simulink model "leda_actual_1s"  (Simulink 7.4)
settings: Solver=ode45  StartTime=0.0  StopTime=50000  RelTol=1e-3
undefined symbols (not defined in the file): pfb_coeff_gen_calc
libraries: xbsIndex_r4 x5755, casper_library_misc x176, casper_library_delays x48, xps_library x39, xbsReplacements_r4 x4, casper_library_ffts_twiddle x2, simulink x1
(part 1 of 84)
settings Model: Creator=jkocz  LastModifiedBy=jkocz

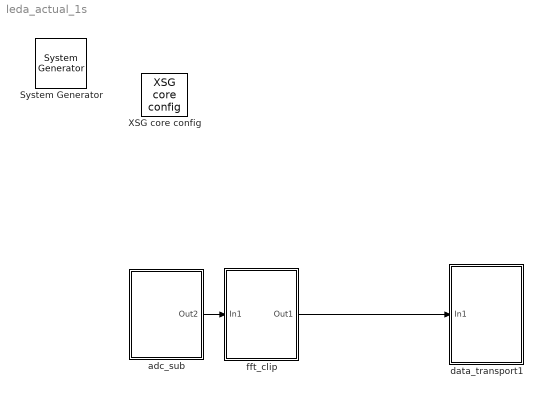
== system leda_actual_1s ==
  " System Generator" : xbsIndex_r4/ System Generator  Tag=genX  SystemSampleTime=-1  xilinxfamily=virtex5  part=xc5vsx95t  speed=-1  package=ff1136  synthesis_tool=XST  clock_wrapper=Clock Enables  directory=./leda_4096_32_test/sysgen  testbench=off  simulink_period=1  sysclk_period=5  dcm_input_clock_period=100  incr_netlist=off  trim_vbits=Everywhere in SubSystem  dbl_ovrd=According to Block Masks  core_generation=According to Block Masks  run_coregen=off  deprecated_control=off  eval_field=0  block_type=sysgen
      settings: block_version=11.4
  XSG core config : xps_library/XSG core config  Tag=xps:xsg  SystemSampleTime=-1  hw_sys=ROACH2:sx475t  clk_src=aux_clk  ROACH_clk_src=adc0_clk  ROACH2_clk_src=aux_clk  clk_rate=200  sample_period=1  synthesis_tool=XST
  adc_sub : Subsystem
  data_transport1 : Subsystem
  fft_clip : Subsystem
  wires:
  fft_clip/1(Out1) -> data_transport1/1(In1)
  adc_sub/1(Out2) -> fft_clip/1(In1)

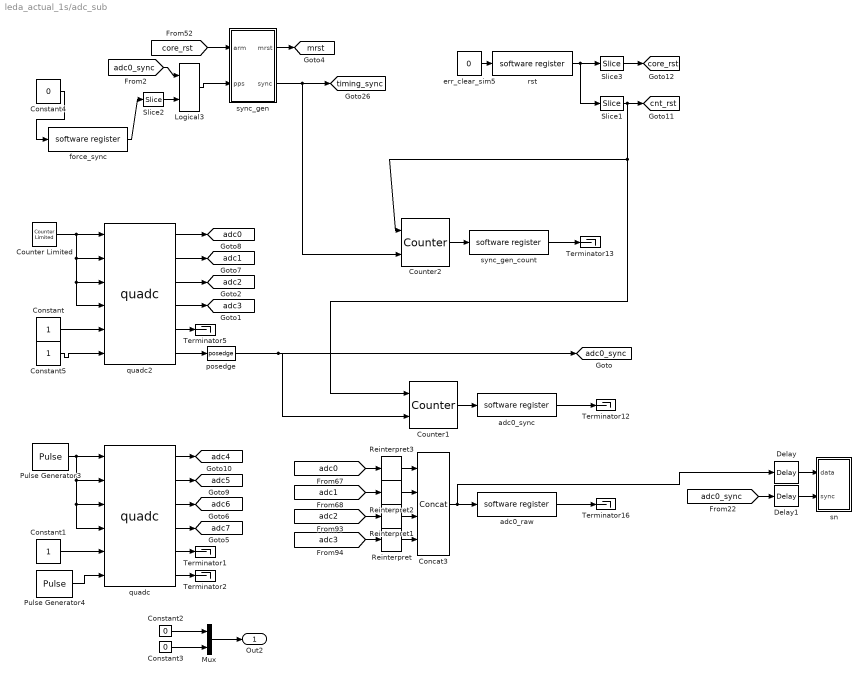
== system leda_actual_1s/adc_sub ==
  Concat3 : xbsIndex_r4/Concat  num_inputs=4  dbl_ovrd=off  block_type=concat
      settings: block_version=10.1.3
  Constant : Constant  OutDataType=sfix(16)  OutScaling=2^0  SampleTime=1/4
  Constant1 : Constant  OutDataType=sfix(16)  OutScaling=2^0  SampleTime=1/4
  Constant2 : Constant  Value=0
  Constant3 : Constant  Value=0
  Constant4 : Constant  Value=0  OutDataType=sfix(16)  OutScaling=2^0  SampleTime=1/4
  Constant5 : Constant  OutDataType=sfix(16)  OutScaling=2^0  SampleTime=1/4
  Counter Limited : simulink/Sources/Counter Limited  SystemSampleTime=-1  uplimit=4095  tsamp=-1
  Counter1 : xbsIndex_r4/Counter  cnt_type=Free Running  cnt_to=Inf  operation=Up  start_count=0  cnt_by_val=1  arith_type=Unsigned  n_bits=64  bin_pt=0  load_pin=off  rst=on  en=on  explicit_period=on  period=1  dbl_ovrd=off  use_behavioral_HDL=off  implementation=Fabric  block_type=counter
      settings: block_version=11.4
  Counter2 : xbsIndex_r4/Counter  cnt_type=Free Running  cnt_to=Inf  operation=Up  start_count=0  cnt_by_val=1  arith_type=Unsigned  n_bits=64  bin_pt=0  load_pin=off  rst=on  en=on  explicit_period=on  period=1  dbl_ovrd=off  use_behavioral_HDL=off  implementation=Fabric  block_type=counter
      settings: block_version=11.4
  Delay : xbsIndex_r4/Delay  en=off  latency=2  dbl_ovrd=off  reg_retiming=off  block_type=delay
      settings: block_version=11.4
  Delay1 : xbsIndex_r4/Delay  en=off  latency=5  dbl_ovrd=off  reg_retiming=off  block_type=delay
      settings: block_version=11.4
  Logical3 : xbsIndex_r4/Logical  logical_function=OR  inputs=2  en=off  latency=0  precision=Full  arith_type=Unsigned  n_bits=16  bin_pt=0  align_bp=on  dbl_ovrd=off  block_type=logical
  Mux : Mux  Inputs=2
  Pulse Generator3 : DiscretePulseGenerator  Amplitude=0.75  Period=2^4  PulseWidth=1  PhaseDelay=9+13+2^8  SampleTime=1/4
  Pulse Generator4 : DiscretePulseGenerator  Amplitude=1  Period=2^16  PulseWidth=1  PhaseDelay=0  SampleTime=1
  Reinterpret{,1..3} (4 alike) : xbsIndex_r4/Reinterpret  force_arith_type=on  arith_type=Unsigned  force_bin_pt=on  bin_pt=0  block_type=reinterpret
      settings: infoedit="Changes signal type without altering the binary representation.   You can changed the signal between signed and unsigned, and relocate the binary point.<br><br>Hardware notes: In hardware this block costs nothing.<br><br>Example:  Suppose the input is 6 bits wide, signed, with 2 fractional bits, and the output is forced to unsigned with 0 fractional bits.  Then an input of -2.0 (1110.00 in binary 2's complement) becomes an output of 56 (111000 in binary)."
  Slice1 : xbsIndex_r4/Slice  nbits=1  boolean_output=on  mode=Lower Bit Location + Width  bit1=0  base1=MSB of Input  bit0=0  base0=LSB of Input  dbl_ovrd=off  block_type=slice
      settings: block_version=10.1.3
  Slice2 : xbsIndex_r4/Slice  nbits=1  boolean_output=on  mode=Lower Bit Location + Width  bit1=0  base1=MSB of Input  bit0=0  base0=LSB of Input  dbl_ovrd=off  block_type=slice
      settings: block_version=10.1.3
  Slice3 : xbsIndex_r4/Slice  nbits=1  boolean_output=on  mode=Lower Bit Location + Width  bit1=0  base1=MSB of Input  bit0=1  base0=LSB of Input  dbl_ovrd=off  block_type=slice
      settings: block_version=10.1.3
  Terminator{1..2,5,12..13,16} (6 alike) : Terminator
  adc0_raw : xps_library/software register  Tag=xps:sw_reg  SystemSampleTime=-1  io_dir=To Processor  arith_type=Unsigned  bitwidth=32  bin_pt=0  sample_period=1  gw_name=leda_actual_1s_adc_sub_adc0_raw_user_data_in
  adc0_sync : xps_library/software register  Tag=xps:sw_reg  SystemSampleTime=-1  io_dir=To Processor  arith_type=Unsigned  bitwidth=32  bin_pt=0  sample_period=1  gw_name=leda_actual_1s_adc_sub_adc0_sync_user_data_in
  err_clear_sim5 : Constant  Value=0  OutDataType=sfix(16)  OutScaling=2^0
  force_sync : xps_library/software register  Tag=xps:sw_reg  SystemSampleTime=-1  io_dir=From Processor  arith_type=Unsigned  bitwidth=32  bin_pt=0  sample_period=1  gw_name=leda_actual_1s_adc_sub_force_sync_user_data_out
      link override r_10gbe_demo_gbe_core_rst_user_data_out: arith_type=Unsigned  n_bits=32  bin_pt=0  period=1
      link override r_10gbe_test_cnt_rst_user_data_out: arith_type=Unsigned  n_bits=32  bin_pt=0  period=1
      link override r_10gbe_test_gbe_core_rst_user_data_out: arith_type=Unsigned  n_bits=32  bin_pt=0  period=1
      link override r_xaui_test_cnt_rst_user_data_out: arith_type=Unsigned  n_bits=32  bin_pt=0  period=1
  posedge : casper_library_misc/posedge  Tag=Rising Edge Detector  SystemSampleTime=-1
  quadc : xps_library/quadc  Tag=xps:quadc  SystemSampleTime=-1  adc_brd=0  adc_clk_rate=200
  quadc2 : xps_library/quadc  Tag=xps:quadc  SystemSampleTime=-1  adc_brd=0  adc_clk_rate=200
  rst : xps_library/software register  Tag=xps:sw_reg  SystemSampleTime=-1  io_dir=From Processor  arith_type=Unsigned  bitwidth=32  bin_pt=0  sample_period=1  gw_name=leda_actual_1s_adc_sub_rst_user_data_out
      link override r_10gbe_demo_gbe_core_rst_user_data_out: arith_type=Unsigned  n_bits=32  bin_pt=0  period=1
      link override r_10gbe_test_cnt_rst_user_data_out: arith_type=Unsigned  n_bits=32  bin_pt=0  period=1
      link override r_10gbe_test_gbe_core_rst_user_data_out: arith_type=Unsigned  n_bits=32  bin_pt=0  period=1
      link override r_xaui_test_cnt_rst_user_data_out: arith_type=Unsigned  n_bits=32  bin_pt=0  period=1
  sn : Subsystem
  sync_gen : Subsystem  mask(sync_gen_hw){sync_period_bits=27}
      mask prompts: sync_period_bits "Size of sync generator counter (bits)"
      mask help:
      | This circuit implements a scheme for synchronizing a design to within an FPGA clock of an external 1 pulse-per-second signal.
      |
      | * An arm pulse will cause the sync_gen to stop issuing sync pulses.
      | * It will then ignore the next input trigger (1PPS), while sync is flushed through the DSP pipeline.
      | * It will then lock onto the next trigger (1PPS) and issue syncs thereafter every sync_period clocks.
      | * You will need a multiple of (pfb_fir taps*LCM(FFT reorder orders) *fft bins/parallel inputs). See the sync memo for further details.
  sync_gen_count : xps_library/software register  Tag=xps:sw_reg  SystemSampleTime=-1  io_dir=To Processor  arith_type=Unsigned  bitwidth=32  bin_pt=0  sample_period=1  gw_name=leda_actual_1s_adc_sub_sync_gen_count_user_data_in
  Out2 : Outport  OutDataType=sfix(16)  OutScaling=2^0
  tag scope: adc0=global, adc0_sync=global, adc1=global, adc2=global, adc3=global, adc4=global, adc5=global, adc6=global, adc7=global, cnt_rst=global, core_rst=global, mrst=global, timing_sync=global
  wires:
  sync_gen/1(mrst) -> [mrst]
  sync_gen/2(sync) -> [timing_sync], Counter2/2
  Logical3/1 -> sync_gen/2(pps)
  [core_rst] -> sync_gen/1(arm)
  quadc2/1 -> [adc0]
  Counter Limited/1 -> quadc2/4, quadc2/3, quadc2/2, quadc2/1
  quadc2/2 -> [adc1]
  quadc2/3 -> [adc2]
  quadc2/4 -> [adc3]
  quadc2/5 -> Terminator5/1
  quadc2/6 -> posedge/1
  Constant/1 -> quadc2/5
  quadc/1 -> [adc4]
  Pulse Generator3/1 -> quadc/4, quadc/3, quadc/2, quadc/1
  quadc/2 -> [adc5]
  quadc/3 -> [adc6]
  quadc/4 -> [adc7]
  quadc/5 -> Terminator1/1
  quadc/6 -> Terminator2/1
  Constant1/1 -> quadc/5
  Pulse Generator4/1 -> quadc/6
  [adc0_sync] -> Logical3/1
  Mux/1 -> Out2/1
  Constant2/1 -> Mux/1
  Constant3/1 -> Mux/2
  err_clear_sim5/1 -> rst/1
  rst/1 -> Slice3/1, Slice1/1
  Slice1/1 -> [cnt_rst], Counter1/1, Counter2/1
  Slice3/1 -> [core_rst]
  Constant5/1 -> quadc2/6
  force_sync/1 -> Slice2/1
  Slice2/1 -> Logical3/2
  Constant4/1 -> force_sync/1
  adc0_raw/1 -> Terminator16/1
  adc0_sync/1 -> Terminator12/1
  Counter1/1 -> adc0_sync/1
  sync_gen_count/1 -> Terminator13/1
  Counter2/1 -> sync_gen_count/1
  Concat3/1 -> adc0_raw/1, Delay/1
  [adc0] -> Reinterpret3/1
  [adc1] -> Reinterpret2/1
  [adc2] -> Reinterpret1/1
  [adc3] -> Reinterpret/1
  Reinterpret3/1 -> Concat3/1
  Reinterpret2/1 -> Concat3/2
  Reinterpret1/1 -> Concat3/3
  Reinterpret/1 -> Concat3/4
  posedge/1 -> Counter1/2, [adc0_sync]
  [adc0_sync] -> Delay1/1
  Delay1/1 -> sn/2(sync)
  Delay/1 -> sn/1(data)

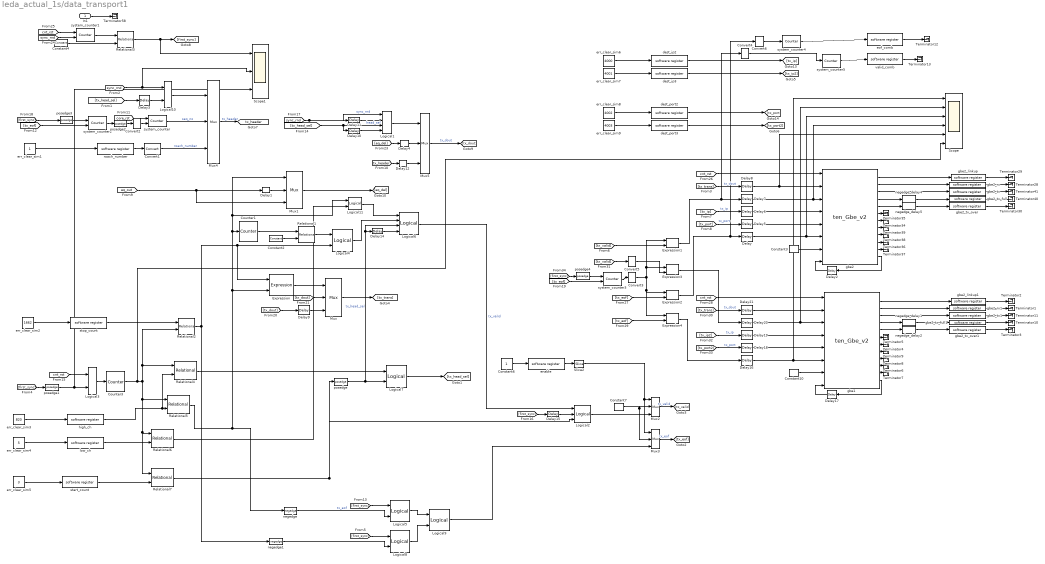
== system leda_actual_1s/data_transport1 ==
  In1 : Inport  OutDataType=sfix(16)  OutScaling=2^0
  Constant10 : xbsIndex_r4/Constant  arith_type=Boolean  const=1  n_bits=1  bin_pt=0  explicit_period=on  period=1  equ=P=C  opselect=C  inp2=PCIN>>17  opr=+  inp1=P  carry=CIN  dbl_ovrd=off  block_type=constant
  Constant2 : xbsIndex_r4/Constant  arith_type=Unsigned  const=25  n_bits=11  bin_pt=0  explicit_period=on  period=1  equ=P=C  opselect=C  inp2=PCIN>>17  opr=+  inp1=P  carry=CIN  dbl_ovrd=off  block_type=constant
  Constant3 : xbsIndex_r4/Constant  arith_type=Boolean  const=1  n_bits=1  bin_pt=0  explicit_period=on  period=1  equ=P=C  opselect=C  inp2=PCIN>>17  opr=+  inp1=P  carry=CIN  dbl_ovrd=off  block_type=constant
  Constant4 : xbsIndex_r4/Constant  arith_type=Unsigned  const=1  n_bits=2  bin_pt=0  explicit_period=on  period=1  equ=P=C  opselect=C  inp2=PCIN>>17  opr=+  inp1=P  carry=CIN  dbl_ovrd=off  block_type=constant
  Constant6 : Constant  OutDataType=sfix(16)  OutScaling=2^0
  Constant7 : xbsIndex_r4/Constant  arith_type=Boolean  const=0  n_bits=1  bin_pt=0  explicit_period=on  period=1  equ=P=C  opselect=C  inp2=PCIN>>17  opr=+  inp1=P  carry=CIN  dbl_ovrd=off  block_type=constant
  Convert1 : xbsIndex_r4/Convert  arith_type=Unsigned  n_bits=64  bin_pt=0  quantization=Truncate  overflow=Wrap  latency=0  dbl_ovrd=off  pipeline=off  block_type=convert
      settings: block_version=11.4
  Convert{2..6} (5 alike) : xbsIndex_r4/Convert  arith_type=Boolean  n_bits=64  bin_pt=0  quantization=Truncate  overflow=Wrap  latency=0  dbl_ovrd=off  pipeline=off  block_type=convert
      settings: block_version=11.4
  Counter1 : xbsIndex_r4/Counter  cnt_type=Free Running  cnt_to=1023  operation=Up  start_count=0  cnt_by_val=1  arith_type=Unsigned  n_bits=13  bin_pt=0  load_pin=off  rst=on  en=off  explicit_period=on  period=1  dbl_ovrd=off  use_behavioral_HDL=off  implementation=Fabric  use_rpm=on  block_type=counter
      settings: xl_area=[0, 0, 0, 0, 0, 0, 0]
  Counter3 : xbsIndex_r4/Counter  cnt_type=Free Running  cnt_to=1023  operation=Up  start_count=0  cnt_by_val=1  arith_type=Unsigned  n_bits=13  bin_pt=0  load_pin=off  rst=on  en=off  explicit_period=on  period=1  dbl_ovrd=off  use_behavioral_HDL=off  implementation=Fabric  use_rpm=on  block_type=counter
      settings: xl_area=[0, 0, 0, 0, 0, 0, 0]
  Delay{,3,7,16,20} (5 alike) : xbsIndex_r4/Delay  en=off  latency=0  dbl_ovrd=off  reg_retiming=off  block_type=delay
      settings: block_version=11.4
  Delay1 : xbsIndex_r4/Delay  en=off  latency=2  dbl_ovrd=off  reg_retiming=on  block_type=delay
      settings: xl_area=[0, 0, 0, 0, 0, 0, 0]
  Delay{5..6,8,10..11,14,18..19,21} (9 alike) : xbsIndex_r4/Delay  en=off  latency=1  dbl_ovrd=off  reg_retiming=off  block_type=delay
      settings: block_version=11.4
  Delay12 : xbsIndex_r4/Delay  en=off  latency=1  dbl_ovrd=off  reg_retiming=on  block_type=delay
      settings: xl_area=[0, 0, 0, 0, 0, 0, 0]
  Delay15 : xbsIndex_r4/Delay  en=off  latency=3  dbl_ovrd=off  reg_retiming=off  block_type=delay
      settings: block_version=11.4
  Delay17 : xbsIndex_r4/Delay  en=off  latency=1  dbl_ovrd=off  reg_retiming=on  block_type=delay
  Delay2 : xbsIndex_r4/Delay  en=off  latency=1  dbl_ovrd=off  reg_retiming=on  block_type=delay
  Delay4 : xbsIndex_r4/Delay  en=off  latency=0  dbl_ovrd=off  reg_retiming=on  block_type=delay
      settings: xl_area=[0, 0, 0, 0, 0, 0, 0]
  Delay9 : xbsIndex_r4/Delay  en=off  latency=26  dbl_ovrd=off  reg_retiming=off  block_type=delay
      settings: block_version=11.4
  Expression : xbsIndex_r4/Expression  expression=a & ~b  align_bp=on  en=off  latency=0  precision=Full  arith_type=Unsigned  n_bits=16  bin_pt=0  dbl_ovrd=off  block_type=expr
      settings: block_version=11.4
  Expression1 : xbsIndex_r4/Expression  expression=a & b  align_bp=on  en=off  latency=0  precision=User Defined  arith_type=Unsigned  n_bits=1  bin_pt=0  dbl_ovrd=off  block_type=expr
      settings: block_version=11.4
  Expression2 : xbsIndex_r4/Expression  expression=a & b  align_bp=on  en=off  latency=0  precision=User Defined  arith_type=Unsigned  n_bits=1  bin_pt=0  dbl_ovrd=off  block_type=expr
      settings: block_version=11.4
  Expression3 : xbsIndex_r4/Expression  expression=~a & b  align_bp=on  en=off  latency=0  precision=User Defined  arith_type=Unsigned  n_bits=1  bin_pt=0  dbl_ovrd=off  block_type=expr
      settings: block_version=11.4
  Expression4 : xbsIndex_r4/Expression  expression=~a & b  align_bp=on  en=off  latency=0  precision=User Defined  arith_type=Unsigned  n_bits=1  bin_pt=0  dbl_ovrd=off  block_type=expr
      settings: block_version=11.4
  Logical1 : xbsIndex_r4/Logical  logical_function=OR  inputs=4  en=off  latency=0  precision=Full  arith_type=Unsigned  n_bits=16  bin_pt=0  align_bp=on  dbl_ovrd=off  block_type=logical
  Logical{3,7,9..10} (4 alike) : xbsIndex_r4/Logical  logical_function=OR  inputs=2  en=off  latency=0  precision=Full  arith_type=Unsigned  n_bits=16  bin_pt=0  align_bp=on  dbl_ovrd=off  block_type=logical
      settings: block_version=11.4
  Logical{4..5,8,11} (4 alike) : xbsIndex_r4/Logical  logical_function=AND  inputs=2  en=off  latency=0  precision=Full  arith_type=Unsigned  n_bits=16  bin_pt=0  align_bp=on  dbl_ovrd=off  block_type=logical
      settings: block_version=11.4
  Logical2 : xbsIndex_r4/Logical  logical_function=AND  inputs=3  en=off  latency=0  precision=Full  arith_type=Unsigned  n_bits=16  bin_pt=0  align_bp=on  dbl_ovrd=off  block_type=logical
      settings: block_version=11.4
  Logical6 : xbsIndex_r4/Logical  logical_function=OR  inputs=4  en=off  latency=1  precision=Full  arith_type=Unsigned  n_bits=16  bin_pt=0  align_bp=on  dbl_ovrd=off  block_type=logical
      settings: block_version=11.4
  Mux : xbsIndex_r4/Mux  inputs=2  en=off  latency=1  precision=Full  arith_type=Unsigned  n_bits=16  bin_pt=14  quantization=Truncate  overflow=Wrap  dbl_ovrd=off  block_type=mux
      settings: block_version=11.4
  Mux1 : xbsIndex_r4/Mux  inputs=2  en=off  latency=0  precision=Full  arith_type=Unsigned  n_bits=16  bin_pt=14  quantization=Truncate  overflow=Wrap  dbl_ovrd=off  block_type=mux
      settings: block_version=11.4
  Mux2 : xbsIndex_r4/Mux  inputs=2  en=off  latency=1  precision=Full  arith_type="Signed  (2's comp)"  n_bits=8  bin_pt=2  quantization=Truncate  overflow=Wrap  dbl_ovrd=off  block_type=mux
      settings: xl_area=[0, 0, 0, 0, 0, 0, 0]
  Mux3 : xbsIndex_r4/Mux  inputs=2  en=off  latency=1  precision=Full  arith_type="Signed  (2's comp)"  n_bits=8  bin_pt=2  quantization=Truncate  overflow=Wrap  dbl_ovrd=off  block_type=mux
      settings: xl_area=[0, 0, 0, 0, 0, 0, 0]
  Mux4 : xbsIndex_r4/Mux  inputs=2  en=off  latency=0  precision=Full  arith_type="Signed  (2's comp)"  n_bits=8  bin_pt=2  quantization=Truncate  overflow=Wrap  dbl_ovrd=off  block_type=mux
      settings: xl_area=[0, 0, 0, 0, 0, 0, 0]
  Mux5 : xbsIndex_r4/Mux  inputs=2  en=off  latency=0  precision=Full  arith_type="Signed  (2's comp)"  n_bits=8  bin_pt=2  quantization=Truncate  overflow=Wrap  dbl_ovrd=off  block_type=mux
      settings: xl_area=[0, 0, 0, 0, 0, 0, 0]
  Relational1 : xbsIndex_r4/Relational  mode=a>b  en=off  latency=0  dbl_ovrd=off  block_type=relational
      settings: xl_area=[0, 0, 0, 0, 0, 0, 0]  block_version=10.1.3
  Relational2 : xbsIndex_r4/Relational  mode=a>b  en=off  latency=1  dbl_ovrd=off  block_type=relational
      settings: xl_area=[0, 0, 0, 0, 0, 0, 0]  block_version=10.1.3
  Relational3 : xbsIndex_r4/Relational  mode=a>=b  en=off  latency=0  dbl_ovrd=off  block_type=relational
      settings: xl_area=[0, 0, 0, 0, 0, 0, 0]  block_version=10.1.3
  Relational4 : xbsIndex_r4/Relational  mode=a=b  en=off  latency=1  dbl_ovrd=off  block_type=relational
      settings: xl_area=[0, 0, 0, 0, 0, 0, 0]  block_version=10.1.3
  Relational5 : xbsIndex_r4/Relational  mode=a<b  en=off  latency=1  dbl_ovrd=off  block_type=relational
      settings: xl_area=[0, 0, 0, 0, 0, 0, 0]  block_version=10.1.3
  Relational6 : xbsIndex_r4/Relational  mode=a>b  en=off  latency=1  dbl_ovrd=off  block_type=relational
      settings: xl_area=[0, 0, 0, 0, 0, 0, 0]  block_version=10.1.3
  Relational7 : xbsIndex_r4/Relational  mode=a>b  en=off  latency=1  dbl_ovrd=off  block_type=relational
      settings: xl_area=[0, 0, 0, 0, 0, 0, 0]  block_version=10.1.3
  Scope : Scope  NumInputPorts=6
      settings: YMin=-5~-5~-5~-5~0~840  YMax=5~5~5~5~5~850
  Scope1 : Scope  NumInputPorts=3
      settings: YMin=-5~-5~-5  YMax=5~5~5
  Slice2 : xbsIndex_r4/Slice  nbits=1  boolean_output=off  mode=Lower Bit Location + Width  bit1=1  base1=MSB of Input  bit0=0  base0=LSB of Input  dbl_ovrd=off  block_type=slice
      settings: block_version=10.1.3
  Terminator{1..13,28..30,34..41,58} (25 alike) : Terminator
  dest_ip2 : xps_library/software register  Tag=xps:sw_reg  SystemSampleTime=-1  io_dir=From Processor  arith_type=Unsigned  bitwidth=32  bin_pt=0  sample_period=1  gw_name=leda_actual_1s_data_transport1_dest_ip2_user_data_out
      link override r_10gbe_demo_dest_ip_user_data_out: arith_type=Unsigned  n_bits=32  bin_pt=0  period=1
      link override r_10gbe_test_dest_ip_user_data_out: arith_type=Unsigned  n_bits=32  bin_pt=0  period=1
      link override r_xaui_test_cnt_rst_user_data_out: arith_type=Unsigned  n_bits=32  bin_pt=0  period=1
  dest_ip3 : xps_library/software register  Tag=xps:sw_reg  SystemSampleTime=-1  io_dir=From Processor  arith_type=Unsigned  bitwidth=32  bin_pt=0  sample_period=1  gw_name=leda_actual_1s_data_transport1_dest_ip3_user_data_out
      link override r_10gbe_demo_dest_ip_user_data_out: arith_type=Unsigned  n_bits=32  bin_pt=0  period=1
      link override r_10gbe_test_dest_ip_user_data_out: arith_type=Unsigned  n_bits=32  bin_pt=0  period=1
      link override r_xaui_test_cnt_rst_user_data_out: arith_type=Unsigned  n_bits=32  bin_pt=0  period=1
  dest_port2 : xps_library/software register  Tag=xps:sw_reg  SystemSampleTime=-1  io_dir=From Processor  arith_type=Unsigned  bitwidth=32  bin_pt=0  sample_period=1  gw_name=leda_actual_1s_data_transport1_dest_port2_user_data_out
      link override r_10gbe_demo_dest_port_user_data_out: arith_type=Unsigned  n_bits=32  bin_pt=0  period=1
      link override r_10gbe_test_dest_port_user_data_out: arith_type=Unsigned  n_bits=32  bin_pt=0  period=1
      link override r_xaui_test_cnt_rst_user_data_out: arith_type=Unsigned  n_bits=32  bin_pt=0  period=1
  dest_port3 : xps_library/software register  Tag=xps:sw_reg  SystemSampleTime=-1  io_dir=From Processor  arith_type=Unsigned  bitwidth=32  bin_pt=0  sample_period=1  gw_name=leda_actual_1s_data_transport1_dest_port3_user_data_out
      link override r_10gbe_demo_dest_port_user_data_out: arith_type=Unsigned  n_bits=32  bin_pt=0  period=1
      link override r_10gbe_test_dest_port_user_data_out: arith_type=Unsigned  n_bits=32  bin_pt=0  period=1
      link override r_xaui_test_cnt_rst_user_data_out: arith_type=Unsigned  n_bits=32  bin_pt=0  period=1
  enable : xps_library/software register  Tag=xps:sw_reg  SystemSampleTime=-1  io_dir=From Processor  arith_type=Unsigned  bitwidth=32  bin_pt=0  sample_period=1  gw_name=leda_actual_1s_data_transport1_enable_user_data_out
      link override r_10gbe_demo_pkt_sim_run_user_data_out: arith_type=Unsigned  n_bits=32  bin_pt=0  period=1
      link override r_10gbe_test_pkt_sim_run_user_data_out: arith_type=Unsigned  n_bits=32  bin_pt=0  period=1
      link override test_pkt_buffer_10_1_p_mux_up_run_user_data_out: arith_type=Unsigned  n_bits=32  bin_pt=0  period=1
  eof_comb : xps_library/software register  Tag=xps:sw_reg  SystemSampleTime=-1  io_dir=To Processor  arith_type=Unsigned  bitwidth=32  bin_pt=0  sample_period=1  gw_name=leda_actual_1s_data_transport1_eof_comb_user_data_in
      link override r_10gbe_demo_dest_ip_user_data_out: arith_type=Unsigned  n_bits=32  bin_pt=0  period=1
      link override r_10gbe_test_dest_ip_user_data_out: arith_type=Unsigned  n_bits=32  bin_pt=0  period=1
      link override r_xaui_test_cnt_rst_user_data_out: arith_type=Unsigned  n_bits=32  bin_pt=0  period=1
  err_clear_sim1 : Constant  OutDataType=sfix(16)  OutScaling=2^0
  err_clear_sim2 : Constant  Value=1662  OutDataType=sfix(16)  OutScaling=2^0
  err_clear_sim3 : Constant  Value=820  OutDataType=sfix(16)  OutScaling=2^0
  err_clear_sim4 : Constant  Value=5  OutDataType=sfix(16)  OutScaling=2^0
  err_clear_sim5 : Constant  Value=3  OutDataType=sfix(16)  OutScaling=2^0
  err_clear_sim6 : Constant  Value=4000  OutDataType=sfix(16)  OutScaling=2^0
  err_clear_sim7 : Constant  Value=4001  OutDataType=sfix(16)  OutScaling=2^0
  err_clear_sim8 : Constant  Value=4002  OutDataType=sfix(16)  OutScaling=2^0
  err_clear_sim9 : Constant  Value=4003  OutDataType=sfix(16)  OutScaling=2^0
  gbe1 : xps_library/ten_Gbe_v2  Tag=xps:tengbe_v2  SystemSampleTime=-1  flavour=cx4  slot=1  port_r1=0  port_r2_cx4=0  port_r2_sfpp=0  rx_dist_ram=off  large_frames=on  show_param=on  pre_emph_r2=0.74  pre_emph=3  post_emph_r2=0.18  rxeqmix_r2=7  swing_r2=940  swing=800  fab_en=off  fab_mac=hex2dec('123456789105')  fab_ip=192*(2^24) + 168*(2^16) + 6*(2^8) + 15*(2^0)  fab_udp=10002  fab_gate=192*(2^24) + 168*(2^16) + 6*(2^8) + 0  cpu_rx_en=on  cpu_tx_en=on
  gbe2 : xps_library/ten_Gbe_v2  Tag=xps:tengbe_v2  SystemSampleTime=-1  flavour=cx4  slot=1  port_r1=0  port_r2_cx4=0  port_r2_sfpp=0  rx_dist_ram=off  large_frames=on  show_param=on  pre_emph_r2=0.74  pre_emph=3  post_emph_r2=0.18  rxeqmix_r2=7  swing_r2=940  swing=800  fab_en=off  fab_mac=hex2dec('123456789100')  fab_ip=192*(2^24) + 168*(2^16) + 5*(2^8) + 14*(2^0)  fab_udp=10002  fab_gate=192*(2^24) + 168*(2^16) + 5*(2^8) + 0  cpu_rx_en=on  cpu_tx_en=on
  gbe2_linkup : xps_library/software register  Tag=xps:sw_reg  SystemSampleTime=-1  io_dir=To Processor  arith_type=Unsigned  bitwidth=32  bin_pt=0  sample_period=1  gw_name=leda_actual_1s_data_transport1_gbe2_linkup_user_data_in
      link override convert: arith_type=Unsigned  n_bits=32  bin_pt=0
  gbe2_linkup1 : xps_library/software register  Tag=xps:sw_reg  SystemSampleTime=-1  io_dir=To Processor  arith_type=Unsigned  bitwidth=32  bin_pt=0  sample_period=1  gw_name=leda_actual_1s_data_transport1_gbe2_linkup1_user_data_in
      link override convert: arith_type=Unsigned  n_bits=32  bin_pt=0
  gbe2_rx : xps_library/software register  Tag=xps:sw_reg  SystemSampleTime=-1  io_dir=To Processor  arith_type=Unsigned  bitwidth=32  bin_pt=0  sample_period=1  gw_name=leda_actual_1s_data_transport1_gbe2_rx_user_data_in
      link override convert: arith_type=Unsigned  n_bits=32  bin_pt=0
  gbe2_rx1 : xps_library/software register  Tag=xps:sw_reg  SystemSampleTime=-1  io_dir=To Processor  arith_type=Unsigned  bitwidth=32  bin_pt=0  sample_period=1  gw_name=leda_actual_1s_data_transport1_gbe2_rx1_user_data_in
      link override convert: arith_type=Unsigned  n_bits=32  bin_pt=0
  gbe2_tx : xps_library/software register  Tag=xps:sw_reg  SystemSampleTime=-1  io_dir=To Processor  arith_type=Unsigned  bitwidth=32  bin_pt=0  sample_period=1  gw_name=leda_actual_1s_data_transport1_gbe2_tx_user_data_in
      link override convert: arith_type=Unsigned  n_bits=32  bin_pt=0
  gbe2_tx1 : xps_library/software register  Tag=xps:sw_reg  SystemSampleTime=-1  io_dir=To Processor  arith_type=Unsigned  bitwidth=32  bin_pt=0  sample_period=1  gw_name=leda_actual_1s_data_transport1_gbe2_tx1_user_data_in
      link override convert: arith_type=Unsigned  n_bits=32  bin_pt=0
  gbe2_tx_full : xps_library/software register  Tag=xps:sw_reg  SystemSampleTime=-1  io_dir=To Processor  arith_type=Unsigned  bitwidth=32  bin_pt=0  sample_period=1  gw_name=leda_actual_1s_data_transport1_gbe2_tx_full_user_data_in
      link override convert: arith_type=Unsigned  n_bits=32  bin_pt=0
  gbe2_tx_full1 : xps_library/software register  Tag=xps:sw_reg  SystemSampleTime=-1  io_dir=To Processor  arith_type=Unsigned  bitwidth=32  bin_pt=0  sample_period=1  gw_name=leda_actual_1s_data_transport1_gbe2_tx_full1_user_data_in
      link override convert: arith_type=Unsigned  n_bits=32  bin_pt=0
  gbe2_tx_over : xps_library/software register  Tag=xps:sw_reg  SystemSampleTime=-1  io_dir=To Processor  arith_type=Unsigned  bitwidth=32  bin_pt=0  sample_period=1  gw_name=leda_actual_1s_data_transport1_gbe2_tx_over_user_data_in
      link override convert: arith_type=Unsigned  n_bits=32  bin_pt=0
  gbe2_tx_over1 : xps_library/software register  Tag=xps:sw_reg  SystemSampleTime=-1  io_dir=To Processor  arith_type=Unsigned  bitwidth=32  bin_pt=0  sample_period=1  gw_name=leda_actual_1s_data_transport1_gbe2_tx_over1_user_data_in
      link override convert: arith_type=Unsigned  n_bits=32  bin_pt=0
  high_ch : xps_library/software register  Tag=xps:sw_reg  SystemSampleTime=-1  io_dir=From Processor  arith_type=Unsigned  bitwidth=32  bin_pt=0  sample_period=1  gw_name=leda_actual_1s_data_transport1_high_ch_user_data_out
      link override r_10gbe_demo_dest_ip_user_data_out: arith_type=Unsigned  n_bits=32  bin_pt=0  period=1
      link override r_10gbe_test_dest_ip_user_data_out: arith_type=Unsigned  n_bits=32  bin_pt=0  period=1
      link override r_xaui_test_cnt_rst_user_data_out: arith_type=Unsigned  n_bits=32  bin_pt=0  period=1
  low_ch : xps_library/software register  Tag=xps:sw_reg  SystemSampleTime=-1  io_dir=From Processor  arith_type=Unsigned  bitwidth=32  bin_pt=0  sample_period=1  gw_name=leda_actual_1s_data_transport1_low_ch_user_data_out
      link override r_10gbe_demo_dest_ip_user_data_out: arith_type=Unsigned  n_bits=32  bin_pt=0  period=1
      link override r_10gbe_test_dest_ip_user_data_out: arith_type=Unsigned  n_bits=32  bin_pt=0  period=1
      link override r_xaui_test_cnt_rst_user_data_out: arith_type=Unsigned  n_bits=32  bin_pt=0  period=1
  negedge : casper_library_misc/negedge  Tag=Rising Edge Detector  SystemSampleTime=-1
  negedge1 : casper_library_misc/negedge  Tag=Rising Edge Detector  SystemSampleTime=-1
  negedge_delay{1..2,4..5} (4 alike) : casper_library_misc/negedge_delay  SystemSampleTime=-1  pulse_len=2^20
  posedge{,1..4} (5 alike) : casper_library_misc/posedge  Tag=Rising Edge Detector  SystemSampleTime=-1
  roach_number : xps_library/software register  Tag=xps:sw_reg  SystemSampleTime=-1  io_dir=From Processor  arith_type=Unsigned  bitwidth=32  bin_pt=0  sample_period=1  gw_name=leda_actual_1s_data_transport1_roach_number_user_data_out
      link override leda_f_8input_r3_data_transport1_roach_number_user_data_out: arith_type=Unsigned  n_bits=32  bin_pt=0  period=1
      link override leda_f_8input_r4_data_transport1_roach_number_user_data_out: arith_type=Unsigned  n_bits=32  bin_pt=0  period=1
      link override r_10gbe_demo_dest_ip_user_data_out: arith_type=Unsigned  n_bits=32  bin_pt=0  period=1
      link override r_10gbe_test_dest_ip_user_data_out: arith_type=Unsigned  n_bits=32  bin_pt=0  period=1
      link override r_xaui_test_cnt_rst_user_data_out: arith_type=Unsigned  n_bits=32  bin_pt=0  period=1
  start_count : xps_library/software register  Tag=xps:sw_reg  SystemSampleTime=-1  io_dir=From Processor  arith_type=Unsigned  bitwidth=32  bin_pt=0  sample_period=1  gw_name=leda_actual_1s_data_transport1_start_count_user_data_out
      link override r_10gbe_demo_dest_ip_user_data_out: arith_type=Unsigned  n_bits=32  bin_pt=0  period=1
      link override r_10gbe_test_dest_ip_user_data_out: arith_type=Unsigned  n_bits=32  bin_pt=0  period=1
      link override r_xaui_test_cnt_rst_user_data_out: arith_type=Unsigned  n_bits=32  bin_pt=0  period=1
  stop_count : xps_library/software register  Tag=xps:sw_reg  SystemSampleTime=-1  io_dir=From Processor  arith_type=Unsigned  bitwidth=32  bin_pt=0  sample_period=1  gw_name=leda_actual_1s_data_transport1_stop_count_user_data_out
      link override r_10gbe_demo_dest_ip_user_data_out: arith_type=Unsigned  n_bits=32  bin_pt=0  period=1
      link override r_10gbe_test_dest_ip_user_data_out: arith_type=Unsigned  n_bits=32  bin_pt=0  period=1
      link override r_xaui_test_cnt_rst_user_data_out: arith_type=Unsigned  n_bits=32  bin_pt=0  period=1
  system_counter : xbsIndex_r4/Counter  cnt_type=Free Running  cnt_to=Inf  operation=Up  start_count=0  cnt_by_val=1  arith_type=Unsigned  n_bits=64  bin_pt=0  load_pin=off  rst=on  en=on  explicit_period=on  period=1  dbl_ovrd=off  use_behavioral_HDL=off  implementation=Fabric  block_type=counter
      settings: block_version=11.4
  system_counter1 : xbsIndex_r4/Counter  cnt_type=Free Running  cnt_to=Inf  operation=Up  start_count=0  cnt_by_val=1  arith_type=Unsigned  n_bits=64  bin_pt=0  load_pin=off  rst=on  en=on  explicit_period=on  period=1  dbl_ovrd=off  use_behavioral_HDL=off  implementation=Fabric  block_type=counter
      settings: block_version=11.4
  system_counter2 : xbsIndex_r4/Counter  cnt_type=Free Running  cnt_to=Inf  operation=Up  start_count=1  cnt_by_val=1  arith_type=Unsigned  n_bits=1  bin_pt=0  load_pin=off  rst=on  en=on  explicit_period=on  period=1  dbl_ovrd=off  use_behavioral_HDL=off  implementation=Fabric  block_type=counter
      settings: block_version=11.4
  system_counter3 : xbsIndex_r4/Counter  cnt_type=Free Running  cnt_to=Inf  operation=Up  start_count=0  cnt_by_val=1  arith_type=Unsigned  n_bits=1  bin_pt=0  load_pin=off  rst=on  en=on  explicit_period=on  period=1  dbl_ovrd=off  use_behavioral_HDL=off  implementation=Fabric  block_type=counter
      settings: block_version=11.4
  system_counter4 : xbsIndex_r4/Counter  cnt_type=Free Running  cnt_to=Inf  operation=Up  start_count=0  cnt_by_val=1  arith_type=Unsigned  n_bits=64  bin_pt=0  load_pin=off  rst=off  en=on  explicit_period=on  period=1  dbl_ovrd=off  use_behavioral_HDL=off  implementation=Fabric  block_type=counter
      settings: block_version=11.4
  system_counter5 : xbsIndex_r4/Counter  cnt_type=Free Running  cnt_to=Inf  operation=Up  start_count=0  cnt_by_val=1  arith_type=Unsigned  n_bits=64  bin_pt=0  load_pin=off  rst=off  en=on  explicit_period=on  period=1  dbl_ovrd=off  use_behavioral_HDL=off  implementation=Fabric  block_type=counter
      settings: block_version=11.4
  valid_comb : xps_library/software register  Tag=xps:sw_reg  SystemSampleTime=-1  io_dir=To Processor  arith_type=Unsigned  bitwidth=32  bin_pt=0  sample_period=1  gw_name=leda_actual_1s_data_transport1_valid_comb_user_data_in
      link override r_10gbe_demo_dest_port_user_data_out: arith_type=Unsigned  n_bits=32  bin_pt=0  period=1
      link override r_10gbe_test_dest_port_user_data_out: arith_type=Unsigned  n_bits=32  bin_pt=0  period=1
      link override r_xaui_test_cnt_rst_user_data_out: arith_type=Unsigned  n_bits=32  bin_pt=0  period=1
  tag scope: tx_header=global
  wires:
  Constant3/1 -> gbe2/7
  [tx_port] -> Delay5/1  "tx_port"
  gbe2/12 -> Delay2/1
  Delay2/1 -> gbe2/8
  gbe2/5 -> negedge_delay5/1
  gbe2/1 -> gbe2_linkup/1
  gbe2/2 -> gbe2_rx/1
  gbe2/3 -> gbe2_tx/1
  gbe2/4 -> negedge_delay4/1
  negedge_delay5/1 -> gbe2_tx_over/1
  negedge_delay4/1 -> gbe2_tx_full/1
  gbe2/9 -> Terminator38/1
  gbe2_linkup/1 -> Terminator29/1
  gbe2_rx/1 -> Terminator28/1
  gbe2_tx/1 -> Terminator41/1
  gbe2_tx_full/1 -> Terminator40/1
  gbe2_tx_over/1 -> Terminator30/1
  gbe2/6 -> Terminator35/1
  gbe2/7 -> Terminator34/1
  gbe2/8 -> Terminator39/1
  gbe2/10 -> Terminator36/1
  gbe2/11 -> Terminator37/1
  In1/1 -> Terminator58/1
  negedge/1 -> Logical5/2  "tx_eof"
  Relational5/1 -> negedge/1, Expression/2, Counter1/1, Mux1/1, Logical11/1
  Counter3/1 -> Relational4/1, Relational2/2, Relational5/1, Relational6/1, Relational7/1, Scope/6
  high_ch/1 -> Relational5/2, Relational4/2
  [tx_trans] -> Delay8/1  "tx_dout"
  [tx_ip] -> Delay6/1  "tx_ip"
  [tx_header] -> Delay12/1
  system_counter/1 -> Mux4/2  "seq_no"
  roach_number/1 -> Convert1/1
  [core_rst] -> system_counter/1
  err_clear_sim1/1 -> roach_number/1
  Mux5/1 -> [tx_dout]  "tx_dout"
  Delay4/1 -> Mux5/2
  posedge/1 -> Logical7/2, Delay14/1, Logical6/3  "tx_head_sel"
  Relational2/1 -> Expression/1, Logical4/2, negedge1/1
  [sync_rnd] -> Delay11/1, Logical1/1  "sync_rnd"
  Logical1/1 -> Mux5/1
  Delay/1 -> gbe2/6, Scope/3
  Delay5/1 -> gbe2/5
  Delay6/1 -> gbe2/4
  Delay7/1 -> gbe2/3, Scope/4
  Delay8/1 -> gbe2/2, Scope/5
  Expression/1 -> Mux/1
  [tx_dout] -> Mux/2
  [tx_dout] -> Delay9/1
  Mux/1 -> [tx_trans]
  Delay9/1 -> Mux/3
  Mux1/1 -> [eq_del]
  [eq_del] -> Delay4/1
  Delay1/1 -> Mux1/2
  Counter1/1 -> Relational1/1
  Constant2/1 -> Relational1/2
  Relational1/1 -> Logical4/1
  system_counter1/1 -> Relational3/1
  Constant4/1 -> Relational3/2
  Relational3/1 -> [first_sync], Scope1/1
  Logical7/1 -> [tx_head_sel]
  Relational4/1 -> Logical7/1
  negedge1/1 -> Logical8/2
  Logical5/1 -> Logical9/1
  Logical8/1 -> Logical9/2
  Logical9/1 -> Mux3/3
  [cnt_rst] -> gbe2/1
  Constant7/1 -> Mux2/2, Mux3/2
  enable/1 -> Slice2/1
  Constant6/1 -> enable/1
  Slice2/1 -> Mux2/1, Mux3/1
  Expression1/1 -> Delay7/1, Convert4/1
  Expression2/1 -> Delay/1, Convert6/1
  Mux2/1 -> [tx_valid]  "tx_valid"
  Mux3/1 -> [tx_eof]  "tx_eof"
  Convert1/1 -> Mux4/3  "roach_number"
  Mux4/1 -> [tx_header]  "tx_header"
  [tx_head_sel] -> Delay3/1
  Logical6/1 -> Logical2/1  "tx_valid"
  Logical2/1 -> Mux2/3
  Logical3/1 -> Counter3/1
  [cnt_rst] -> Logical3/1
  posedge1/1 -> Logical3/2, Scope1/3
  [sync_rnd] -> Logical10/1, Scope1/2
  Logical10/1 -> Mux4/1
  Delay3/1 -> Logical10/2
  [tx_head_sel] -> Delay10/1, Logical1/3  "head_sel"
  Delay10/1 -> Logical1/4
  Delay11/1 -> Logical1/2
  [first_sync] -> posedge1/1
  [first_sync] -> Logical8/1
  [first_sync] -> Logical5/1
  [first_sync] -> Delay15/1
  Delay12/1 -> Mux5/3
  system_counter2/1 -> posedge2/1
  posedge2/1 -> Convert2/1
  [first_sync] -> posedge3/1
  [tx_eof] -> system_counter2/2
  Convert2/1 -> system_counter/2
  posedge3/1 -> system_counter2/1
  dest_port2/1 -> [tx_port]
  dest_port3/1 -> [tx_port2]
  err_clear_sim6/1 -> dest_ip2/1
  err_clear_sim7/1 -> dest_ip3/1
  err_clear_sim8/1 -> dest_port2/1
  err_clear_sim9/1 -> dest_port3/1
  Relational6/1 -> Logical11/2
  Logical11/1 -> Logical6/1
  Relational7/1 -> posedge/1, Logical2/3
  Delay15/1 -> Logical2/2
  Logical4/1 -> Logical6/2
  Delay14/1 -> Logical6/4
  Constant10/1 -> gbe1/7
  [tx_port2] -> Delay18/1  "tx_port"
  gbe1/12 -> Delay17/1
  Delay17/1 -> gbe1/8
  gbe1/5 -> negedge_delay2/1
  gbe1/1 -> gbe2_linkup1/1
  gbe1/2 -> gbe2_rx1/1
  gbe1/3 -> gbe2_tx1/1
  gbe1/4 -> negedge_delay1/1
  negedge_delay2/1 -> gbe2_tx_over1/1
  negedge_delay1/1 -> gbe2_tx_full1/1
  gbe1/9 -> Terminator8/1
  gbe2_linkup1/1 -> Terminator2/1
  gbe2_rx1/1 -> Terminator1/1
  gbe2_tx1/1 -> Terminator11/1
  gbe2_tx_full1/1 -> Terminator10/1
  gbe2_tx_over1/1 -> Terminator3/1
  gbe1/6 -> Terminator5/1
  gbe1/7 -> Terminator4/1
  gbe1/8 -> Terminator9/1
  gbe1/10 -> Terminator6/1
  gbe1/11 -> Terminator7/1
  [tx_trans] -> Delay21/1  "tx_dout"
  [tx_ip2] -> Delay19/1  "tx_ip"
  Delay16/1 -> gbe1/6, Scope/1
  Delay18/1 -> gbe1/5
  Delay19/1 -> gbe1/4
  Delay20/1 -> gbe1/3, Scope/2
  Delay21/1 -> gbe1/2
  [cnt_rst] -> gbe1/1
  Expression3/1 -> Delay20/1
  Expression4/1 -> Delay16/1
  dest_ip2/1 -> [tx_ip]
  dest_ip3/1 -> [tx_ip2]
  [tx_eof] -> system_counter3/2
  system_counter3/1 -> Convert3/1
  [tx_valid] -> Expression1/2
  [tx_eof] -> Expression2/2
  [tx_valid] -> Convert5/1
  [tx_eof] -> Expression4/2
  Convert3/1 -> Expression1/1, Expression3/1, Expression2/1, Expression4/1
  Convert5/1 -> Expression3/2
  system_counter4/1 -> eof_comb/1
  system_counter5/1 -> valid_comb/1
  Convert4/1 -> system_counter5/1
  eof_comb/1 -> Terminator12/1
  valid_comb/1 -> Terminator13/1
  Convert6/1 -> system_counter4/1
  [cnt_rst] -> system_counter1/1
  [sync_rnd] -> system_counter1/2
  low_ch/1 -> Relational6/2
  [first_sync] -> posedge4/1
  posedge4/1 -> system_counter3/1
  err_clear_sim2/1 -> stop_count/1
  err_clear_sim3/1 -> high_ch/1
  err_clear_sim4/1 -> low_ch/1
  err_clear_sim5/1 -> start_count/1
  stop_count/1 -> Relational2/1
  start_count/1 -> Relational7/2
  [eq_out] -> Delay1/1, Mux1/3
